simulink model "cddDcmApp"  (R2022b) (continued)
== system cddDcmApp == (continued)
  CarCfgForClimaCtrl_CarCfgForAirMtrlSnsr/1 -> cddDcmApp_20ms_Runnable_sys/34(CarCfgForClimaCtrl_CarCfgForAirMtrlSnsr_read)
  CarCfgForClimaCtrl_CarCfgForAirQly/1 -> cddDcmApp_20ms_Runnable_sys/35(CarCfgForClimaCtrl_CarCfgForAirQly_read)
  CarCfgForClimaCtrl_CarCfgForAmntOfSeat/1 -> cddDcmApp_20ms_Runnable_sys/36(CarCfgForClimaCtrl_CarCfgForAmntOfSeat_read)
  CarCfgForClimaCtrl_CarCfgForAutDemst/1 -> cddDcmApp_20ms_Runnable_sys/37(CarCfgForClimaCtrl_CarCfgForAutDemst_read)
  CarCfgForClimaCtrl_CarCfgForAuxWtrPmp/1 -> cddDcmApp_20ms_Runnable_sys/38(CarCfgForClimaCtrl_CarCfgForAuxWtrPmp_read)
  CarCfgForClimaCtrl_CarCfgForBRAND/1 -> cddDcmApp_20ms_Runnable_sys/39(CarCfgForClimaCtrl_CarCfgForBRAND_read)
  CarCfgForClimaCtrl_CarCfgForBypVlvInHvac/1 -> cddDcmApp_20ms_Runnable_sys/40(CarCfgForClimaCtrl_CarCfgForBypVlvInHvac_read)
  CarCfgForClimaCtrl_CarCfgForCabinOxgenSupply/1 -> cddDcmApp_20ms_Runnable_sys/41(CarCfgForClimaCtrl_CarCfgForCabinOxgenSupply_read)
  CarCfgForClimaCtrl_CarCfgForClsVlvInHvac/1 -> cddDcmApp_20ms_Runnable_sys/42(CarCfgForClimaCtrl_CarCfgForClsVlvInHvac_read)
  CarCfgForClimaCtrl_CarCfgForCmprAcTyp/1 -> cddDcmApp_20ms_Runnable_sys/43(CarCfgForClimaCtrl_CarCfgForCmprAcTyp_read)
  CarCfgForClimaCtrl_CarCfgForCmptmtClng/1 -> cddDcmApp_20ms_Runnable_sys/44(CarCfgForClimaCtrl_CarCfgForCmptmtClng_read)
  CarCfgForClimaCtrl_CarCfgForElectricLiftingModule/1 -> cddDcmApp_20ms_Runnable_sys/45(CarCfgForClimaCtrl_CarCfgForElectricLiftingModule_read)
  CarCfgForClimaCtrl_CarCfgForElecVentAdjmt/1 -> cddDcmApp_20ms_Runnable_sys/46(CarCfgForClimaCtrl_CarCfgForElecVentAdjmt_read)
  CarCfgForClimaCtrl_CarCfgForExtVentnClimaCtrl/1 -> cddDcmApp_20ms_Runnable_sys/47(CarCfgForClimaCtrl_CarCfgForExtVentnClimaCtrl_read)
  CarCfgForClimaCtrl_CarCfgForFlcTyp/1 -> cddDcmApp_20ms_Runnable_sys/48(CarCfgForClimaCtrl_CarCfgForFlcTyp_read)
  CarCfgForClimaCtrl_CarCfgForFloatingDriving/1 -> cddDcmApp_20ms_Runnable_sys/49(CarCfgForClimaCtrl_CarCfgForFloatingDriving_read)
  CarCfgForClimaCtrl_CarCfgForFoldCSD/1 -> cddDcmApp_20ms_Runnable_sys/50(CarCfgForClimaCtrl_CarCfgForFoldCSD_read)
  CarCfgForClimaCtrl_CarCfgForFuTyp/1 -> cddDcmApp_20ms_Runnable_sys/51(CarCfgForClimaCtrl_CarCfgForFuTyp_read)
  CarCfgForClimaCtrl_CarCfgForGlassTyp/1 -> cddDcmApp_20ms_Runnable_sys/52(CarCfgForClimaCtrl_CarCfgForGlassTyp_read)
  CarCfgForClimaCtrl_CarCfgForHeadRadar/1 -> cddDcmApp_20ms_Runnable_sys/53(CarCfgForClimaCtrl_CarCfgForHeadRadar_read)
  CarCfgForClimaCtrl_CarCfgForHeatPmp/1 -> cddDcmApp_20ms_Runnable_sys/54(CarCfgForClimaCtrl_CarCfgForHeatPmp_read)
  CarCfgForClimaCtrl_CarCfgForHeatrDurgDrvg/1 -> cddDcmApp_20ms_Runnable_sys/55(CarCfgForClimaCtrl_CarCfgForHeatrDurgDrvg_read)
  CarCfgForClimaCtrl_CarCfgForHeatrTyp/1 -> cddDcmApp_20ms_Runnable_sys/56(CarCfgForClimaCtrl_CarCfgForHeatrTyp_read)
  CarCfgForClimaCtrl_CarCfgForHvacFilTyp/1 -> cddDcmApp_20ms_Runnable_sys/57(CarCfgForClimaCtrl_CarCfgForHvacFilTyp_read)
  CarCfgForClimaCtrl_CarCfgForHvacFlrSnsrForT/1 -> cddDcmApp_20ms_Runnable_sys/58(CarCfgForClimaCtrl_CarCfgForHvacFlrSnsrForT_read)
  CarCfgForClimaCtrl_CarCfgForHvacHeatrSnsrT/1 -> cddDcmApp_20ms_Runnable_sys/59(CarCfgForClimaCtrl_CarCfgForHvacHeatrSnsrT_read)
  CarCfgForClimaCtrl_CarCfgForHvacRe/1 -> cddDcmApp_20ms_Runnable_sys/60(CarCfgForClimaCtrl_CarCfgForHvacRe_read)
  CarCfgForClimaCtrl_CarCfgForHvacTyp/1 -> cddDcmApp_20ms_Runnable_sys/61(CarCfgForClimaCtrl_CarCfgForHvacTyp_read)
  CarCfgForClimaCtrl_CarCfgForInteriorViewCamera/1 -> cddDcmApp_20ms_Runnable_sys/62(CarCfgForClimaCtrl_CarCfgForInteriorViewCamera_read)
  CarCfgForClimaCtrl_CarCfgForLifeDetection/1 -> cddDcmApp_20ms_Runnable_sys/63(CarCfgForClimaCtrl_CarCfgForLifeDetection_read)
  CarCfgForClimaCtrl_CarCfgForLoVoltElecCfg/1 -> cddDcmApp_20ms_Runnable_sys/64(CarCfgForClimaCtrl_CarCfgForLoVoltElecCfg_read)
  CarCfgForClimaCtrl_CarCfgForNavSysandIntnmap/1 -> cddDcmApp_20ms_Runnable_sys/65(CarCfgForClimaCtrl_CarCfgForNavSysandIntnmap_read)
  CarCfgForClimaCtrl_CarCfgForNrOfDoor/1 -> cddDcmApp_20ms_Runnable_sys/66(CarCfgForClimaCtrl_CarCfgForNrOfDoor_read)
  CarCfgForClimaCtrl_CarCfgForPipeInFuTank/1 -> cddDcmApp_20ms_Runnable_sys/67(CarCfgForClimaCtrl_CarCfgForPipeInFuTank_read)
  CarCfgForClimaCtrl_CarCfgForPowerSeats/1 -> cddDcmApp_20ms_Runnable_sys/68(CarCfgForClimaCtrl_CarCfgForPowerSeats_read)
  CarCfgForClimaCtrl_CarCfgForPreClng/1 -> cddDcmApp_20ms_Runnable_sys/69(CarCfgForClimaCtrl_CarCfgForPreClng_read)
  CarCfgForClimaCtrl_CarCfgForPrkgCDSNotAvl/1 -> cddDcmApp_20ms_Runnable_sys/70(CarCfgForClimaCtrl_CarCfgForPrkgCDSNotAvl_read)
  CarCfgForClimaCtrl_CarCfgForPrkgClima/1 -> cddDcmApp_20ms_Runnable_sys/71(CarCfgForClimaCtrl_CarCfgForPrkgClima_read)
  CarCfgForClimaCtrl_CarCfgForPrpsnTyp/1 -> cddDcmApp_20ms_Runnable_sys/72(CarCfgForClimaCtrl_CarCfgForPrpsnTyp_read)
  CarCfgForClimaCtrl_CarCfgForPTCInFrontHVAC/1 -> cddDcmApp_20ms_Runnable_sys/73(CarCfgForClimaCtrl_CarCfgForPTCInFrontHVAC_read)
  CarCfgForClimaCtrl_CarCfgForRemEngStrtPreCdn/1 -> cddDcmApp_20ms_Runnable_sys/74(CarCfgForClimaCtrl_CarCfgForRemEngStrtPreCdn_read)
  CarCfgForClimaCtrl_CarCfgForRoofHatch/1 -> cddDcmApp_20ms_Runnable_sys/75(CarCfgForClimaCtrl_CarCfgForRoofHatch_read)
  CarCfgForClimaCtrl_CarCfgForSeatHeatgCal/1 -> cddDcmApp_20ms_Runnable_sys/76(CarCfgForClimaCtrl_CarCfgForSeatHeatgCal_read)
  CarCfgForClimaCtrl_CarCfgForSeatHeatgForRowFirst/1 -> cddDcmApp_20ms_Runnable_sys/77(CarCfgForClimaCtrl_CarCfgForSeatHeatgForRowFirst_read)
  CarCfgForClimaCtrl_CarCfgForSeatHeatgForRowSec/1 -> cddDcmApp_20ms_Runnable_sys/78(CarCfgForClimaCtrl_CarCfgForSeatHeatgForRowSec_read)
  CarCfgForClimaCtrl_CarCfgForSeatVentnForRowFirst/1 -> cddDcmApp_20ms_Runnable_sys/79(CarCfgForClimaCtrl_CarCfgForSeatVentnForRowFirst_read)
  CarCfgForClimaCtrl_CarCfgForSeatVentnForRowSec/1 -> cddDcmApp_20ms_Runnable_sys/80(CarCfgForClimaCtrl_CarCfgForSeatVentnForRowSec_read)
  CarCfgForClimaCtrl_CarCfgForSecRowVentnCenter/1 -> cddDcmApp_20ms_Runnable_sys/81(CarCfgForClimaCtrl_CarCfgForSecRowVentnCenter_read)
  CarCfgForClimaCtrl_CarCfgForSecRowVentnSide/1 -> cddDcmApp_20ms_Runnable_sys/82(CarCfgForClimaCtrl_CarCfgForSecRowVentnSide_read)
  CarCfgForClimaCtrl_CarCfgForSmallFlowCyc/1 -> cddDcmApp_20ms_Runnable_sys/83(CarCfgForClimaCtrl_CarCfgForSmallFlowCyc_read)
  CarCfgForClimaCtrl_CarCfgForSmkSnsr/1 -> cddDcmApp_20ms_Runnable_sys/84(CarCfgForClimaCtrl_CarCfgForSmkSnsr_read)
  CarCfgForClimaCtrl_CarCfgForSnsrForCmptmtT/1 -> cddDcmApp_20ms_Runnable_sys/85(CarCfgForClimaCtrl_CarCfgForSnsrForCmptmtT_read)
  CarCfgForClimaCtrl_CarCfgForSnsrHumPrsnt/1 -> cddDcmApp_20ms_Runnable_sys/86(CarCfgForClimaCtrl_CarCfgForSnsrHumPrsnt_read)
  CarCfgForClimaCtrl_CarCfgForStartDevice/1 -> cddDcmApp_20ms_Runnable_sys/87(CarCfgForClimaCtrl_CarCfgForStartDevice_read)
  CarCfgForClimaCtrl_CarCfgForSteerWhlHeatg/1 -> cddDcmApp_20ms_Runnable_sys/88(CarCfgForClimaCtrl_CarCfgForSteerWhlHeatg_read)
  CarCfgForClimaCtrl_CarCfgForSteerWhlPosn/1 -> cddDcmApp_20ms_Runnable_sys/89(CarCfgForClimaCtrl_CarCfgForSteerWhlPosn_read)
  CarCfgForClimaCtrl_CarCfgForSunSnsr/1 -> cddDcmApp_20ms_Runnable_sys/90(CarCfgForClimaCtrl_CarCfgForSunSnsr_read)
  CarCfgForClimaCtrl_CarCfgForTelmNodTyp/1 -> cddDcmApp_20ms_Runnable_sys/91(CarCfgForClimaCtrl_CarCfgForTelmNodTyp_read)
  CarCfgForClimaCtrl_CarCfgForTEMPEREDSTORAGEBOX/1 -> cddDcmApp_20ms_Runnable_sys/92(CarCfgForClimaCtrl_CarCfgForTEMPEREDSTORAGEBOX_read)
  CarCfgForClimaCtrl_CarCfgForThermMngt/1 -> cddDcmApp_20ms_Runnable_sys/93(CarCfgForClimaCtrl_CarCfgForThermMngt_read)
  CarCfgForClimaCtrl_CarCfgForVehTyp/1 -> cddDcmApp_20ms_Runnable_sys/94(CarCfgForClimaCtrl_CarCfgForVehTyp_read)
  CarCfgForClimaCtrl_CarCfgForVentLiftMotorExiSts/1 -> cddDcmApp_20ms_Runnable_sys/95(CarCfgForClimaCtrl_CarCfgForVentLiftMotorExiSts_read)
  CarCfgForClimaCtrl_CarCfgForVgaMotorExistSts/1 -> cddDcmApp_20ms_Runnable_sys/96(CarCfgForClimaCtrl_CarCfgForVgaMotorExistSts_read)
  CarCfgForClimaCtrl_CarCfgForWadingAssistSystem/1 -> cddDcmApp_20ms_Runnable_sys/97(CarCfgForClimaCtrl_CarCfgForWadingAssistSystem_read)
  CarCfgForClimaCtrl_CarCfgForWindHeatd/1 -> cddDcmApp_20ms_Runnable_sys/98(CarCfgForClimaCtrl_CarCfgForWindHeatd_read)
  CarCfgForClimaCtrl_CarCfgWindFollowHumanMov/1 -> cddDcmApp_20ms_Runnable_sys/99(CarCfgForClimaCtrl_CarCfgWindFollowHumanMov_read)
  CarTiGlb_CarTiGlb/1 -> cddDcmApp_20ms_Runnable_sys/100(CarTiGlb_CarTiGlb_read)
  CarTiGlb_CarTiGlb_IsUpdated/1 -> cddDcmApp_20ms_Runnable_sys/101(CarTiGlb_CarTiGlb_IsUpdated_read)
  CcmIntCo2Lvl_CcmIntCo2Lvl/1 -> cddDcmApp_20ms_Runnable_sys/102(CcmIntCo2Lvl_CcmIntCo2Lvl_read)
  CCMLIN1frameSts_CCMLIN1frameSts/1 -> cddDcmApp_20ms_Runnable_sys/103(CCMLIN1frameSts_CCMLIN1frameSts_read)
  CCMLIN2frameSts_CCMLIN2frameSts/1 -> cddDcmApp_20ms_Runnable_sys/104(CCMLIN2frameSts_CCMLIN2frameSts_read)
  CCMLIN3frameSts_CCMLIN3frameSts/1 -> cddDcmApp_20ms_Runnable_sys/105(CCMLIN3frameSts_CCMLIN3frameSts_read)
  CdsFrameSts_CdsFrameSts/1 -> cddDcmApp_20ms_Runnable_sys/106(CdsFrameSts_CdsFrameSts_read)
  ClimaObsActv_ClimaObsActv/1 -> cddDcmApp_20ms_Runnable_sys/107(ClimaObsActv_ClimaObsActv_read)
  CmpmtInAirPmAQI_CmpmtInAirPmAQI/1 -> cddDcmApp_20ms_Runnable_sys/108(CmpmtInAirPmAQI_CmpmtInAirPmAQI_read)
  CmpmtInAirPmDnsty_CmpmtInAirPmDnsty/1 -> cddDcmApp_20ms_Runnable_sys/109(CmpmtInAirPmDnsty_CmpmtInAirPmDnsty_read)
  CmptmtAirTEstimdAtRowFirst_CmptmtAirTEstimdAtRowFirst/1 -> cddDcmApp_20ms_Runnable_sys/110(CmptmtAirTEstimdAtRowFirst_CmptmtAirTEstimdAtRowFirst_read)
  CmptmtAirTEstimdAtRowFirstIni_CmptmtAirTEstimdAtRowFirstIni/1 -> cddDcmApp_20ms_Runnable_sys/111(CmptmtAirTEstimdAtRowFirstIni_CmptmtAirTEstimdAtRowFirstIni_read)
  CmptmtAirTEstimdAtRowSec_CmptmtAirTEstimdAtRowSec/1 -> cddDcmApp_20ms_Runnable_sys/112(CmptmtAirTEstimdAtRowSec_CmptmtAirTEstimdAtRowSec_read)
  CmptmtAirTEstimdAtRowSecIni_CmptmtAirTEstimdAtRowSecIni/1 -> cddDcmApp_20ms_Runnable_sys/113(CmptmtAirTEstimdAtRowSecIni_CmptmtAirTEstimdAtRowSecIni_read)
  CmptmtAirTEstimdFirstNvRamIni_CmptmtAirTEstimdFirstNvRamIni/1 -> cddDcmApp_20ms_Runnable_sys/114(CmptmtAirTEstimdFirstNvRamIni_CmptmtAirTEstimdFirstNvRamIni_read)
  CmptmtAirTEstimdSecNvRamIni_CmptmtAirTEstimdSecNvRamIni/1 -> cddDcmApp_20ms_Runnable_sys/115(CmptmtAirTEstimdSecNvRamIni_CmptmtAirTEstimdSecNvRamIni_read)
  CmptmtFrntWindDewT_CmptmtFrntWindDewT/1 -> cddDcmApp_20ms_Runnable_sys/116(CmptmtFrntWindDewT_CmptmtFrntWindDewT_read)
  CmptmtFrntWindT_CmptmtFrntWindT/1 -> cddDcmApp_20ms_Runnable_sys/117(CmptmtFrntWindT_CmptmtFrntWindT_read)
  CmptmtOxygenConcVluNgcc_CmptmtOxygenConcVluNgcc/1 -> cddDcmApp_20ms_Runnable_sys/118(CmptmtOxygenConcVluNgcc_CmptmtOxygenConcVluNgcc_read)
  CmptmtRelHum_CmptmtRelHum/1 -> cddDcmApp_20ms_Runnable_sys/119(CmptmtRelHum_CmptmtRelHum_read)
  CmptmtRelHum_CmptmtRelHum_IsUpdated/1 -> cddDcmApp_20ms_Runnable_sys/120(CmptmtRelHum_CmptmtRelHum_IsUpdated_read)
  CmptmtTRearRaw_CmptmtTRearRaw/1 -> cddDcmApp_20ms_Runnable_sys/121(CmptmtTRearRaw_CmptmtTRearRaw_read)
  CoupldCtrlErrAtRowFirstLe_CoupldCtrlErrAtRowFirstLe/1 -> cddDcmApp_20ms_Runnable_sys/122(CoupldCtrlErrAtRowFirstLe_CoupldCtrlErrAtRowFirstLe_read)
  CoupldCtrlErrAtRowFirstRi_CoupldCtrlErrAtRowFirstRi/1 -> cddDcmApp_20ms_Runnable_sys/123(CoupldCtrlErrAtRowFirstRi_CoupldCtrlErrAtRowFirstRi_read)
  CoupldCtrlErrAtRowSecLe_CoupldCtrlErrAtRowSecLe/1 -> cddDcmApp_20ms_Runnable_sys/124(CoupldCtrlErrAtRowSecLe_CoupldCtrlErrAtRowSecLe_read)
  CoupldCtrlErrAtRowSecRi_CoupldCtrlErrAtRowSecRi/1 -> cddDcmApp_20ms_Runnable_sys/125(CoupldCtrlErrAtRowSecRi_CoupldCtrlErrAtRowSecRi_read)
  DcMotorCurPosVol_DcMotorCurPosVol/1 -> cddDcmApp_20ms_Runnable_sys/126(DcMotorCurPosVol_DcMotorCurPosVol_read)
  EngT_Ngcc_EngT/1 -> cddDcmApp_20ms_Runnable_sys/127(EngT_Ngcc_EngT_read)
  EvaprTFrntFildForClima_EvaprTFrntFildForClima/1 -> cddDcmApp_20ms_Runnable_sys/128(EvaprTFrntFildForClima_EvaprTFrntFildForClima_read)
  EvaprTReFildForClima_EvaprTReFildForClima/1 -> cddDcmApp_20ms_Runnable_sys/129(EvaprTReFildForClima_EvaprTReFildForClima_read)
  HmiHvacFanLvlFrnt_HmiHvacFanLvlFrnt/1 -> cddDcmApp_20ms_Runnable_sys/130(HmiHvacFanLvlFrnt_HmiHvacFanLvlFrnt_read)
  HmiHvacFanLvlRe_HmiHvacFanLvlRe/1 -> cddDcmApp_20ms_Runnable_sys/131(HmiHvacFanLvlRe_HmiHvacFanLvlRe_read)
  HvacAirTEstimdAtRowFirstLe_HvacAirTEstimdAtRowFirstLe/1 -> cddDcmApp_20ms_Runnable_sys/132(HvacAirTEstimdAtRowFirstLe_HvacAirTEstimdAtRowFirstLe_read)
  HvacAirTEstimdAtRowFirstRi_HvacAirTEstimdAtRowFirstRi/1 -> cddDcmApp_20ms_Runnable_sys/133(HvacAirTEstimdAtRowFirstRi_HvacAirTEstimdAtRowFirstRi_read)
  HvacAirTEstimdAtRowSecLe_HvacAirTEstimdAtRowSecLe/1 -> cddDcmApp_20ms_Runnable_sys/134(HvacAirTEstimdAtRowSecLe_HvacAirTEstimdAtRowSecLe_read)
  HvacAirTEstimdAtRowSecRi_HvacAirTEstimdAtRowSecRi/1 -> cddDcmApp_20ms_Runnable_sys/135(HvacAirTEstimdAtRowSecRi_HvacAirTEstimdAtRowSecRi_read)
  HvacAirTReqAtRowFirstLe_HvacAirTReqAtRowFirstLe/1 -> cddDcmApp_20ms_Runnable_sys/136(HvacAirTReqAtRowFirstLe_HvacAirTReqAtRowFirstLe_read)
  HvacAirTReqAtRowFirstRi_HvacAirTReqAtRowFirstRi/1 -> cddDcmApp_20ms_Runnable_sys/137(HvacAirTReqAtRowFirstRi_HvacAirTReqAtRowFirstRi_read)
  HvacAirTReqAtRowSecLe_HvacAirTReqAtRowSecLe/1 -> cddDcmApp_20ms_Runnable_sys/138(HvacAirTReqAtRowSecLe_HvacAirTReqAtRowSecLe_read)
  HvacAirTReqAtRowSecRi_HvacAirTReqAtRowSecRi/1 -> cddDcmApp_20ms_Runnable_sys/139(HvacAirTReqAtRowSecRi_HvacAirTReqAtRowSecRi_read)
  HvacCfgForClimaCtrl_HvacCfgForAQSTypes/1 -> cddDcmApp_20ms_Runnable_sys/140(HvacCfgForClimaCtrl_HvacCfgForAQSTypes_read)
  HvacCfgForClimaCtrl_HvacCfgForHvacFrontBlowerType/1 -> cddDcmApp_20ms_Runnable_sys/141(HvacCfgForClimaCtrl_HvacCfgForHvacFrontBlowerType_read)
  HvacCfgForClimaCtrl_HvacCfgForHvacLowSideRETXVESts/1 -> cddDcmApp_20ms_Runnable_sys/142(HvacCfgForClimaCtrl_HvacCfgForHvacLowSideRETXVESts_read)
  HvacCfgForClimaCtrl_HvacCfgForHvacMiscExistSts/1 -> cddDcmApp_20ms_Runnable_sys/143(HvacCfgForClimaCtrl_HvacCfgForHvacMiscExistSts_read)
  HvacCfgForClimaCtrl_HvacCfgForHvacMotorExistSts/1 -> cddDcmApp_20ms_Runnable_sys/144(HvacCfgForClimaCtrl_HvacCfgForHvacMotorExistSts_read)
  HvacCfgForClimaCtrl_HvacCfgForHvacReBlowerType/1 -> cddDcmApp_20ms_Runnable_sys/145(HvacCfgForClimaCtrl_HvacCfgForHvacReBlowerType_read)
  HvacCfgForClimaCtrl_HvacCfgForHvacReVentPstn/1 -> cddDcmApp_20ms_Runnable_sys/146(HvacCfgForClimaCtrl_HvacCfgForHvacReVentPstn_read)
  HvacCfgForClimaCtrl_HvacCfgForHvacSensorExistSts/1 -> cddDcmApp_20ms_Runnable_sys/147(HvacCfgForClimaCtrl_HvacCfgForHvacSensorExistSts_read)
  HvacCfgForClimaCtrl_HvacCfgForHvacSnsrRCmptmtESts/1 -> cddDcmApp_20ms_Runnable_sys/148(HvacCfgForClimaCtrl_HvacCfgForHvacSnsrRCmptmtESts_read)
  HvacCfgForClimaCtrl_HvacCfgForHvacSnsrReHexESts/1 -> cddDcmApp_20ms_Runnable_sys/149(HvacCfgForClimaCtrl_HvacCfgForHvacSnsrReHexESts_read)
  HvacFanSet3HvacFanSpd1_HvacFanSet3HvacFanSpd1/1 -> cddDcmApp_20ms_Runnable_sys/150(HvacFanSet3HvacFanSpd1_HvacFanSet3HvacFanSpd1_read)
  HvacFanSts2HvacFanBattI2_HvacFanSts2HvacFanBattI2/1 -> cddDcmApp_20ms_Runnable_sys/151(HvacFanSts2HvacFanBattI2_HvacFanSts2HvacFanBattI2_read)
  HvacFanSts2HvacFanBattU2_HvacFanSts2HvacFanBattU2/1 -> cddDcmApp_20ms_Runnable_sys/152(HvacFanSts2HvacFanBattU2_HvacFanSts2HvacFanBattU2_read)
  HvacFanSts2HvacFanMotU2_HvacFanSts2HvacFanMotU2/1 -> cddDcmApp_20ms_Runnable_sys/153(HvacFanSts2HvacFanMotU2_HvacFanSts2HvacFanMotU2_read)
  HvacFanSts2HvacFanReSts1_HvacFanSts2HvacFanReSts1/1 -> cddDcmApp_20ms_Runnable_sys/154(HvacFanSts2HvacFanReSts1_HvacFanSts2HvacFanReSts1_read)
  HvacFanSts2HvacFanReSts2_HvacFanSts2HvacFanReSts2/1 -> cddDcmApp_20ms_Runnable_sys/155(HvacFanSts2HvacFanReSts2_HvacFanSts2HvacFanReSts2_read)
  HvacFanSts3HvacFanBattI_HvacFanSts3HvacFanBattI/1 -> cddDcmApp_20ms_Runnable_sys/156(HvacFanSts3HvacFanBattI_HvacFanSts3HvacFanBattI_read)
  HvacFanSts3HvacFanBattU_HvacFanSts3HvacFanBattU/1 -> cddDcmApp_20ms_Runnable_sys/157(HvacFanSts3HvacFanBattU_HvacFanSts3HvacFanBattU_read)
  HvacFanSts3HvacFanCircShoOrMotBlkd1_HvacFanSts3HvacFanCircShoOrMotBlkd1/1 -> cddDcmApp_20ms_Runnable_sys/158(HvacFanSts3HvacFanCircShoOrMotBlkd1_HvacFanSts3HvacFanCircShoOrMotBlkd1_read)
  HvacFanSts3HvacFanDiagFlag1_HvacFanSts3HvacFanDiagFlag1/1 -> cddDcmApp_20ms_Runnable_sys/159(HvacFanSts3HvacFanDiagFlag1_HvacFanSts3HvacFanDiagFlag1_read)
  HvacFanSts3HvacFanILimn_HvacFanSts3HvacFanILimn/1 -> cddDcmApp_20ms_Runnable_sys/160(HvacFanSts3HvacFanILimn_HvacFanSts3HvacFanILimn_read)
  HvacFanSts3HvacFanIntErr1_HvacFanSts3HvacFanIntErr1/1 -> cddDcmApp_20ms_Runnable_sys/161(HvacFanSts3HvacFanIntErr1_HvacFanSts3HvacFanIntErr1_read)
  HvacFanSts3HvacFanSpdFd_HvacFanSts3HvacFanSpdFd/1 -> cddDcmApp_20ms_Runnable_sys/162(HvacFanSts3HvacFanSpdFd_HvacFanSts3HvacFanSpdFd_read)
  HvacFanSts3HvacFanTErr_HvacFanSts3HvacFanTErr/1 -> cddDcmApp_20ms_Runnable_sys/163(HvacFanSts3HvacFanTErr_HvacFanSts3HvacFanTErr_read)
  HvacFanSts3HvDetd_HvacFanSts3HvDetd/1 -> cddDcmApp_20ms_Runnable_sys/164(HvacFanSts3HvDetd_HvacFanSts3HvDetd_read)
  HvacFanSts3OverCurrErr_HvacFanSts3OverCurrErr/1 -> cddDcmApp_20ms_Runnable_sys/165(HvacFanSts3OverCurrErr_HvacFanSts3OverCurrErr_read)
  HvacFanSts3UDetdLo_HvacFanSts3UDetdLo/1 -> cddDcmApp_20ms_Runnable_sys/166(HvacFanSts3UDetdLo_HvacFanSts3UDetdLo_read)
  HvacFanVoltReqFrnt_HvacFanVoltReqFrnt/1 -> cddDcmApp_20ms_Runnable_sys/167(HvacFanVoltReqFrnt_HvacFanVoltReqFrnt_read)
  HvacFanVoltReqRe_HvacFanVoltReqRe/1 -> cddDcmApp_20ms_Runnable_sys/168(HvacFanVoltReqRe_HvacFanVoltReqRe_read)
  HvacLpmUFbRe_HvacLpmUFbRe/1 -> cddDcmApp_20ms_Runnable_sys/169(HvacLpmUFbRe_HvacLpmUFbRe_read)
  HvacPwm2MosVFbFrnt_HvacPwm2MosVFbFrnt/1 -> cddDcmApp_20ms_Runnable_sys/170(HvacPwm2MosVFbFrnt_HvacPwm2MosVFbFrnt_read)
  HvacPwmSignErr_HvacPwmSignErr/1 -> cddDcmApp_20ms_Runnable_sys/171(HvacPwmSignErr_HvacPwmSignErr_read)
  InPm25FanErr_InPm25FanErr/1 -> cddDcmApp_20ms_Runnable_sys/172(InPm25FanErr_InPm25FanErr_read)
  InPm25SensrIntErr_InPm25SensrIntErr/1 -> cddDcmApp_20ms_Runnable_sys/173(InPm25SensrIntErr_InPm25SensrIntErr_read)
  InPm25SnrTempErr_InPm25SnrTempErr/1 -> cddDcmApp_20ms_Runnable_sys/174(InPm25SnrTempErr_InPm25SnrTempErr_read)
  InPm25SnrVoltgRangErr_InPm25SnrVoltgRangErr/1 -> cddDcmApp_20ms_Runnable_sys/175(InPm25SnrVoltgRangErr_InPm25SnrVoltgRangErr_read)
  InPm25SnsrElecErr_InPm25SnsrElecErr/1 -> cddDcmApp_20ms_Runnable_sys/176(InPm25SnsrElecErr_InPm25SnsrElecErr_read)
  IntCo2Conc_IntCo2Conc/1 -> cddDcmApp_20ms_Runnable_sys/177(IntCo2Conc_IntCo2Conc_read)
  IntCo2Lvl_IntCo2Lvl/1 -> cddDcmApp_20ms_Runnable_sys/178(IntCo2Lvl_IntCo2Lvl_read)
  LdAdVal_CurrAdVal/1 -> cddDcmApp_20ms_Runnable_sys/179(LdAdVal_CurrAdVal_read)
  LdAdVal_ValidAdVal/1 -> cddDcmApp_20ms_Runnable_sys/180(LdAdVal_ValidAdVal_read)
  MemReadForCenVentLiftModSts_MemReadForCenVentLiftModSts/1 -> cddDcmApp_20ms_Runnable_sys/181(MemReadForCenVentLiftModSts_MemReadForCenVentLiftModSts_read)
  MemReadForClimaEgyMgrForDID_MemReadForClimaEgyMgrForDID/1 -> cddDcmApp_20ms_Runnable_sys/182(MemReadForClimaEgyMgrForDID_MemReadForClimaEgyMgrForDID_read)
  NvMBlockCCMLoadDataArray_NvMBlockCCMLoadDataArray/1 -> cddDcmApp_20ms_Runnable_sys/183(NvMBlockCCMLoadDataArray_NvMBlockCCMLoadDataArray_read)
  NvMBlockDcMtrCalDataArray_NvMBlockDcMtrCalDataArray/1 -> cddDcmApp_20ms_Runnable_sys/184(NvMBlockDcMtrCalDataArray_NvMBlockDcMtrCalDataArray_read)
  ObsvrAirTOffsFromWindTIni_ObsvrAirTOffsFromWindTIni/1 -> cddDcmApp_20ms_Runnable_sys/185(ObsvrAirTOffsFromWindTIni_ObsvrAirTOffsFromWindTIni_read)
  ObsvrCmptmtTIninActv_ObsvrCmptmtTIninActv/1 -> cddDcmApp_20ms_Runnable_sys/186(ObsvrCmptmtTIninActv_ObsvrCmptmtTIninActv_read)
  ObsvrCmptmtTRamRxInt_ObsvrCmptmtTRamRxInt/1 -> cddDcmApp_20ms_Runnable_sys/187(ObsvrCmptmtTRamRxInt_ObsvrCmptmtTRamRxInt_read)
  ObsvrInCarTFrntIni_ObsvrInCarTFrntIni/1 -> cddDcmApp_20ms_Runnable_sys/188(ObsvrInCarTFrntIni_ObsvrInCarTFrntIni_read)
  ObsvrInCarTRearIni_ObsvrInCarTRearIni/1 -> cddDcmApp_20ms_Runnable_sys/189(ObsvrInCarTRearIni_ObsvrInCarTRearIni_read)
  ObsvrIntrMtrlTIni_ObsvrIntrMtrlTIni/1 -> cddDcmApp_20ms_Runnable_sys/190(ObsvrIntrMtrlTIni_ObsvrIntrMtrlTIni_read)
  OGMIntErrNgcc_OGMIntErrNgcc/1 -> cddDcmApp_20ms_Runnable_sys/191(OGMIntErrNgcc_OGMIntErrNgcc_read)
  OGMOvrVoltRangErrNgcc_OGMOvrVoltRangErrNgcc/1 -> cddDcmApp_20ms_Runnable_sys/192(OGMOvrVoltRangErrNgcc_OGMOvrVoltRangErrNgcc_read)
  OGMTempErrNgcc_OGMTempErrNgcc/1 -> cddDcmApp_20ms_Runnable_sys/193(OGMTempErrNgcc_OGMTempErrNgcc_read)
  OGMUndVoltRangErrNgcc_OGMUndVoltRangErrNgcc/1 -> cddDcmApp_20ms_Runnable_sys/194(OGMUndVoltRangErrNgcc_OGMUndVoltRangErrNgcc_read)
  OutdAirQly_OutdAirQly/1 -> cddDcmApp_20ms_Runnable_sys/195(OutdAirQly_OutdAirQly_read)
  OxygenConcSnsrElecErrNgcc_OxygenConcSnsrElecErrNgcc/1 -> cddDcmApp_20ms_Runnable_sys/196(OxygenConcSnsrElecErrNgcc_OxygenConcSnsrElecErrNgcc_read)
  OxygenConcSnsrIntErrNgcc_OxygenConcSnsrIntErrNgcc/1 -> cddDcmApp_20ms_Runnable_sys/197(OxygenConcSnsrIntErrNgcc_OxygenConcSnsrIntErrNgcc_read)
  OxygenConcSnsrOvrVoltRangErrNgcc_OxygenConcSnsrOvrVoltRangErrNgcc/1 -> cddDcmApp_20ms_Runnable_sys/198(OxygenConcSnsrOvrVoltRangErrNgcc_OxygenConcSnsrOvrVoltRangErrNgcc_read)
  OxygenConcSnsrTempErrNgcc_OxygenConcSnsrTempErrNgcc/1 -> cddDcmApp_20ms_Runnable_sys/199(OxygenConcSnsrTempErrNgcc_OxygenConcSnsrTempErrNgcc_read)
  OxygenConcSnsrUndVoltRangErrNgcc_OxygenConcSnsrUndVoltRangErrNgcc/1 -> cddDcmApp_20ms_Runnable_sys/200(OxygenConcSnsrUndVoltRangErrNgcc_OxygenConcSnsrUndVoltRangErrNgcc_read)
  PmsiFrameSts_PmsiFrameSts/1 -> cddDcmApp_20ms_Runnable_sys/201(PmsiFrameSts_PmsiFrameSts_read)
  ReEvapVlvSts_ReEvapVlvSts/1 -> cddDcmApp_20ms_Runnable_sys/202(ReEvapVlvSts_ReEvapVlvSts_read)
  ReHvacActrElecErr_ReHvacActrElecErr/1 -> cddDcmApp_20ms_Runnable_sys/203(ReHvacActrElecErr_ReHvacActrElecErr_read)
  ReHvacActrOvrTempErr_ReHvacActrOvrTempErr/1 -> cddDcmApp_20ms_Runnable_sys/204(ReHvacActrOvrTempErr_ReHvacActrOvrTempErr_read)
  ReHvacActrVoltErr_ReHvacActrVoltErr/1 -> cddDcmApp_20ms_Runnable_sys/205(ReHvacActrVoltErr_ReHvacActrVoltErr_read)
  RelHumSnsrRelHum_RelHumSnsrRelHum/1 -> cddDcmApp_20ms_Runnable_sys/206(RelHumSnsrRelHum_RelHumSnsrRelHum_read)
  RhfaFrameSts_RhfaFrameSts/1 -> cddDcmApp_20ms_Runnable_sys/207(RhfaFrameSts_RhfaFrameSts_read)
  SensorAdValue_SensorAdValue/1 -> cddDcmApp_20ms_Runnable_sys/208(SensorAdValue_SensorAdValue_read)
  SrsAdVal_CurrAdVal/1 -> cddDcmApp_20ms_Runnable_sys/209(SrsAdVal_CurrAdVal_read)
  SrsAdVal_ValidAdVal/1 -> cddDcmApp_20ms_Runnable_sys/210(SrsAdVal_ValidAdVal_read)
  SunData_SunData/1 -> cddDcmApp_20ms_Runnable_sys/211(SunData_SunData_read)
  SWVersion_SWVersion/1 -> cddDcmApp_20ms_Runnable_sys/212(SWVersion_SWVersion_read)
  SysServCarCfgSts_SysServCarID/1 -> cddDcmApp_20ms_Runnable_sys/213(SysServCarCfgSts_SysServCarID_read)
  SysServCarCfgSts_SysServCCPAQSSts/1 -> cddDcmApp_20ms_Runnable_sys/214(SysServCarCfgSts_SysServCCPAQSSts_read)
  SysServCarCfgSts_SysServCCPBGMNum/1 -> cddDcmApp_20ms_Runnable_sys/215(SysServCarCfgSts_SysServCCPBGMNum_read)
  SysServCarCfgSts_SysServCCPHEXSensorSts/1 -> cddDcmApp_20ms_Runnable_sys/216(SysServCarCfgSts_SysServCCPHEXSensorSts_read)
  SysServCarCfgSts_SysServCCPLin2AFUSts/1 -> cddDcmApp_20ms_Runnable_sys/217(SysServCarCfgSts_SysServCCPLin2AFUSts_read)
  SysServCarCfgSts_SysServCCPLin2AIUSts/1 -> cddDcmApp_20ms_Runnable_sys/218(SysServCarCfgSts_SysServCCPLin2AIUSts_read)
  SysServCarCfgSts_SysServCCPLin2AQSSts/1 -> cddDcmApp_20ms_Runnable_sys/219(SysServCarCfgSts_SysServCCPLin2AQSSts_read)
  SysServCarCfgSts_SysServCCPLin2CDSSts/1 -> cddDcmApp_20ms_Runnable_sys/220(SysServCarCfgSts_SysServCCPLin2CDSSts_read)
  SysServCarCfgSts_SysServCCPLin2PMSISts/1 -> cddDcmApp_20ms_Runnable_sys/221(SysServCarCfgSts_SysServCCPLin2PMSISts_read)
  SysServCarCfgSts_SysServCCPLin3VGASts/1 -> cddDcmApp_20ms_Runnable_sys/222(SysServCarCfgSts_SysServCCPLin3VGASts_read)
  SysServCarCfgSts_SysServDUAirVentSnrSts/1 -> cddDcmApp_20ms_Runnable_sys/223(SysServCarCfgSts_SysServDUAirVentSnrSts_read)
  SysServCarCfgSts_SysServDUBlowerPwmSignSts/1 -> cddDcmApp_20ms_Runnable_sys/224(SysServCarCfgSts_SysServDUBlowerPwmSignSts_read)
  SysServCarCfgSts_SysServDUBlowerPwmSignTypeSts/1 -> cddDcmApp_20ms_Runnable_sys/225(SysServCarCfgSts_SysServDUBlowerPwmSignTypeSts_read)
  SysServCarCfgSts_SysServDUBlowerPwmSts/1 -> cddDcmApp_20ms_Runnable_sys/226(SysServCarCfgSts_SysServDUBlowerPwmSts_read)
  SysServCarCfgSts_SysServDUDCDefrostSts/1 -> cddDcmApp_20ms_Runnable_sys/227(SysServCarCfgSts_SysServDUDCDefrostSts_read)
  SysServCarCfgSts_SysServDUDCFLMdeSts/1 -> cddDcmApp_20ms_Runnable_sys/228(SysServCarCfgSts_SysServDUDCFLMdeSts_read)
  SysServCarCfgSts_SysServDUDCModeSts/1 -> cddDcmApp_20ms_Runnable_sys/229(SysServCarCfgSts_SysServDUDCModeSts_read)
  SysServCarCfgSts_SysServDUDCRecSts/1 -> cddDcmApp_20ms_Runnable_sys/230(SysServCarCfgSts_SysServDUDCRecSts_read)
  SysServCarCfgSts_SysServDUDCSModeSts/1 -> cddDcmApp_20ms_Runnable_sys/231(SysServCarCfgSts_SysServDUDCSModeSts_read)
  SysServCarCfgSts_SysServDUDCSTLSts/1 -> cddDcmApp_20ms_Runnable_sys/232(SysServCarCfgSts_SysServDUDCSTLSts_read)
  SysServCarCfgSts_SysServDUDCTLSts/1 -> cddDcmApp_20ms_Runnable_sys/233(SysServCarCfgSts_SysServDUDCTLSts_read)
  SysServCarCfgSts_SysServDUDCTRSts/1 -> cddDcmApp_20ms_Runnable_sys/234(SysServCarCfgSts_SysServDUDCTRSts_read)
  SysServCarCfgSts_SysServDUFLFootSts/1 -> cddDcmApp_20ms_Runnable_sys/235(SysServCarCfgSts_SysServDUFLFootSts_read)
  SysServCarCfgSts_SysServDUFLVentSts/1 -> cddDcmApp_20ms_Runnable_sys/236(SysServCarCfgSts_SysServDUFLVentSts_read)
  SysServCarCfgSts_SysServDUFRFootSts/1 -> cddDcmApp_20ms_Runnable_sys/237(SysServCarCfgSts_SysServDUFRFootSts_read)
  SysServCarCfgSts_SysServDUFRVentSts/1 -> cddDcmApp_20ms_Runnable_sys/238(SysServCarCfgSts_SysServDUFRVentSts_read)
  SysServCarCfgSts_SysServDUIncarMotorSts/1 -> cddDcmApp_20ms_Runnable_sys/239(SysServCarCfgSts_SysServDUIncarMotorSts_read)
  SysServCarCfgSts_SysServDULin1HBMFSts/1 -> cddDcmApp_20ms_Runnable_sys/240(SysServCarCfgSts_SysServDULin1HBMFSts_read)
  SysServCarCfgSts_SysServDULin4HFAFSts/1 -> cddDcmApp_20ms_Runnable_sys/241(SysServCarCfgSts_SysServDULin4HFAFSts_read)
  SysServCarCfgSts_SysServDULin4HFAFStsH/1 -> cddDcmApp_20ms_Runnable_sys/242(SysServCarCfgSts_SysServDULin4HFAFStsH_read)
  SysServCarCfgSts_SysServDUReBlowerPwmSignSts/1 -> cddDcmApp_20ms_Runnable_sys/243(SysServCarCfgSts_SysServDUReBlowerPwmSignSts_read)
  SysServCarCfgSts_SysServDUReBlowerPwmSts/1 -> cddDcmApp_20ms_Runnable_sys/244(SysServCarCfgSts_SysServDUReBlowerPwmSts_read)
  SysServCarCfgSts_SysServDUSFLFootSts/1 -> cddDcmApp_20ms_Runnable_sys/245(SysServCarCfgSts_SysServDUSFLFootSts_read)
  SysServCarCfgSts_SysServDUSFLVentSts/1 -> cddDcmApp_20ms_Runnable_sys/246(SysServCarCfgSts_SysServDUSFLVentSts_read)
  SysServCarCfgSts_SysServDUSFRFootSts/1 -> cddDcmApp_20ms_Runnable_sys/247(SysServCarCfgSts_SysServDUSFRFootSts_read)
  SysServCarCfgSts_SysServDUSFRVentSts/1 -> cddDcmApp_20ms_Runnable_sys/248(SysServCarCfgSts_SysServDUSFRVentSts_read)
  SysServCarCfgSts_SysServDUSnsrForFCmptmtTSts/1 -> cddDcmApp_20ms_Runnable_sys/249(SysServCarCfgSts_SysServDUSnsrForFCmptmtTSts_read)
  SysServCarCfgSts_SysServDUSnsrForRair/1 -> cddDcmApp_20ms_Runnable_sys/250(SysServCarCfgSts_SysServDUSnsrForRair_read)
  SysServCarCfgSts_SysServDUSnsrForRCmptmtTSts/1 -> cddDcmApp_20ms_Runnable_sys/251(SysServCarCfgSts_SysServDUSnsrForRCmptmtTSts_read)
  SysServCarCfgSts_SysServFrntHEXSnsrPosn/1 -> cddDcmApp_20ms_Runnable_sys/252(SysServCarCfgSts_SysServFrntHEXSnsrPosn_read)
  SysServCarCfgSts_SysServLowSideRETXVSts/1 -> cddDcmApp_20ms_Runnable_sys/253(SysServCarCfgSts_SysServLowSideRETXVSts_read)
  SysServCarCfgSts_SysServReHEXSnsrPosn/1 -> cddDcmApp_20ms_Runnable_sys/254(SysServCarCfgSts_SysServReHEXSnsrPosn_read)
  SysServCarCfgSts_SysServRHFASts/1 -> cddDcmApp_20ms_Runnable_sys/255(SysServCarCfgSts_SysServRHFASts_read)
  SysServCarCfgSts_SysServSnsrForFCmptmtTType/1 -> cddDcmApp_20ms_Runnable_sys/256(SysServCarCfgSts_SysServSnsrForFCmptmtTType_read)
  TestRunCriteriaTRC_TestRunCriteriaTRC/1 -> cddDcmApp_20ms_Runnable_sys/257(TestRunCriteriaTRC_TestRunCriteriaTRC_read)
  TiOfDeactvnForClimaObs_TiOfDeactvnForClimaObs/1 -> cddDcmApp_20ms_Runnable_sys/258(TiOfDeactvnForClimaObs_TiOfDeactvnForClimaObs_read)
  VbmrFrameSts_VbmrFrameSts/1 -> cddDcmApp_20ms_Runnable_sys/259(VbmrFrameSts_VbmrFrameSts_read)
  VehBattU_VehBattU/1 -> cddDcmApp_20ms_Runnable_sys/260(VehBattU_VehBattU_read)
  VehBattU_VehBattU_IsUpdated/1 -> cddDcmApp_20ms_Runnable_sys/261(VehBattU_VehBattU_IsUpdated_read)
  VehModMngtGlbSafe1_VehModMngtGlbSafe1/1 -> cddDcmApp_20ms_Runnable_sys/262(VehModMngtGlbSafe1_VehModMngtGlbSafe1_read)
  VehModMngtGlbSafe1_VehModMngtGlbSafe1_IsUpdated/1 -> cddDcmApp_20ms_Runnable_sys/263(VehModMngtGlbSafe1_VehModMngtGlbSafe1_IsUpdated_read)
  VentnActr01ElecErr_VentnActr01ElecErr/1 -> cddDcmApp_20ms_Runnable_sys/264(VentnActr01ElecErr_VentnActr01ElecErr_read)
  VentnActr01OvrTempErr_VentnActr01OvrTempErr/1 -> cddDcmApp_20ms_Runnable_sys/265(VentnActr01OvrTempErr_VentnActr01OvrTempErr_read)
  VentnActr01VoltErr_VentnActr01VoltErr/1 -> cddDcmApp_20ms_Runnable_sys/266(VentnActr01VoltErr_VentnActr01VoltErr_read)
  VentnActr02ElecErr_VentnActr02ElecErr/1 -> cddDcmApp_20ms_Runnable_sys/267(VentnActr02ElecErr_VentnActr02ElecErr_read)
  VentnActr02OvrTempErr_VentnActr02OvrTempErr/1 -> cddDcmApp_20ms_Runnable_sys/268(VentnActr02OvrTempErr_VentnActr02OvrTempErr_read)
  VentnActr02VoltErr_VentnActr02VoltErr/1 -> cddDcmApp_20ms_Runnable_sys/269(VentnActr02VoltErr_VentnActr02VoltErr_read)
  VentnActr03ElecErr_VentnActr03ElecErr/1 -> cddDcmApp_20ms_Runnable_sys/270(VentnActr03ElecErr_VentnActr03ElecErr_read)
  VentnActr03OvrTempErr_VentnActr03OvrTempErr/1 -> cddDcmApp_20ms_Runnable_sys/271(VentnActr03OvrTempErr_VentnActr03OvrTempErr_read)
  VentnActr03VoltErr_VentnActr03VoltErr/1 -> cddDcmApp_20ms_Runnable_sys/272(VentnActr03VoltErr_VentnActr03VoltErr_read)
  VentnActr04ElecErr_VentnActr04ElecErr/1 -> cddDcmApp_20ms_Runnable_sys/273(VentnActr04ElecErr_VentnActr04ElecErr_read)
  VentnActr04OvrTempErr_VentnActr04OvrTempErr/1 -> cddDcmApp_20ms_Runnable_sys/274(VentnActr04OvrTempErr_VentnActr04OvrTempErr_read)
  VentnActr04VoltErr_VentnActr04VoltErr/1 -> cddDcmApp_20ms_Runnable_sys/275(VentnActr04VoltErr_VentnActr04VoltErr_read)
  VentnActr05ElecErr_VentnActr05ElecErr/1 -> cddDcmApp_20ms_Runnable_sys/276(VentnActr05ElecErr_VentnActr05ElecErr_read)
  VentnActr05OvrTempErr_VentnActr05OvrTempErr/1 -> cddDcmApp_20ms_Runnable_sys/277(VentnActr05OvrTempErr_VentnActr05OvrTempErr_read)
  VentnActr05VoltErr_VentnActr05VoltErr/1 -> cddDcmApp_20ms_Runnable_sys/278(VentnActr05VoltErr_VentnActr05VoltErr_read)
  VentnActr06ElecErr_VentnActr06ElecErr/1 -> cddDcmApp_20ms_Runnable_sys/279(VentnActr06ElecErr_VentnActr06ElecErr_read)
  VentnActr06OvrTempErr_VentnActr06OvrTempErr/1 -> cddDcmApp_20ms_Runnable_sys/280(VentnActr06OvrTempErr_VentnActr06OvrTempErr_read)
  VentnActr06VoltErr_VentnActr06VoltErr/1 -> cddDcmApp_20ms_Runnable_sys/281(VentnActr06VoltErr_VentnActr06VoltErr_read)
  VentnActr07ElecErr_VentnActr07ElecErr/1 -> cddDcmApp_20ms_Runnable_sys/282(VentnActr07ElecErr_VentnActr07ElecErr_read)
  VentnActr07OvrTempErr_VentnActr07OvrTempErr/1 -> cddDcmApp_20ms_Runnable_sys/283(VentnActr07OvrTempErr_VentnActr07OvrTempErr_read)
  VentnActr07VoltErr_VentnActr07VoltErr/1 -> cddDcmApp_20ms_Runnable_sys/284(VentnActr07VoltErr_VentnActr07VoltErr_read)
  VentnActr08ElecErr_VentnActr08ElecErr/1 -> cddDcmApp_20ms_Runnable_sys/285(VentnActr08ElecErr_VentnActr08ElecErr_read)
  VentnActr08OvrTempErr_VentnActr08OvrTempErr/1 -> cddDcmApp_20ms_Runnable_sys/286(VentnActr08OvrTempErr_VentnActr08OvrTempErr_read)
  VentnActr08VoltErr_VentnActr08VoltErr/1 -> cddDcmApp_20ms_Runnable_sys/287(VentnActr08VoltErr_VentnActr08VoltErr_read)
  VentnActr09ElecErr_VentnActr09ElecErr/1 -> cddDcmApp_20ms_Runnable_sys/288(VentnActr09ElecErr_VentnActr09ElecErr_read)
  VentnActr09OvrTempErr_VentnActr09OvrTempErr/1 -> cddDcmApp_20ms_Runnable_sys/289(VentnActr09OvrTempErr_VentnActr09OvrTempErr_read)
  VentnActr09VoltErr_VentnActr09VoltErr/1 -> cddDcmApp_20ms_Runnable_sys/290(VentnActr09VoltErr_VentnActr09VoltErr_read)
  BswLifeCycle_LifeCycle/1 -> cddDcmApp_20ms_Runnable_sys/291(BswLifeCycle_LifeCycle_read)
  cddDcmApp_20ms_Runnable/1 -> cddDcmApp_20ms_Runnable_sys/Trigger
  cddDcmApp_Init/1 -> cddDcmApp_Init_sys/Trigger
  Dcm_Reset_Hard/1 -> Dcm_Reset_Hard_sys/Trigger
  Dcm_Reset_JumpToBootLoader/1 -> Dcm_Reset_JumpToBootLoader_sys/Trigger
  DiagSessionCtrl_Default/1 -> DiagSessionCtrl_Default_sys/Trigger
